simulink library "Microgrid_Library"  (R2020a)
settings: Solver=VariableStepAuto  StartTime=0.0  StopTime=10.0  RelTol=1e-3
libraries: simulink x6944, powerlib x1090, simulink_extras x40, powerlib_meascontrol x20
(part 2 of 77)

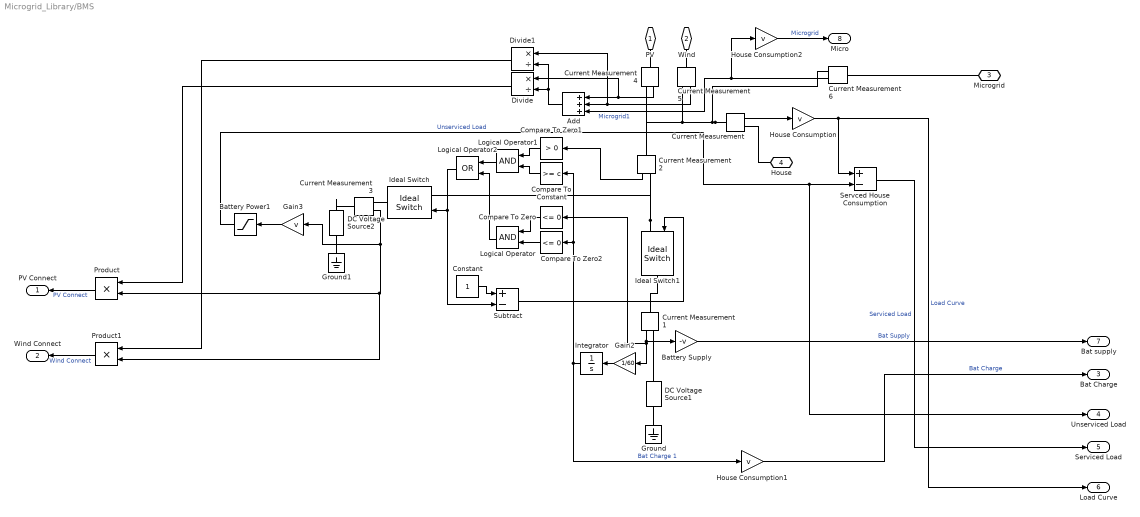
== system Microgrid_Library/BMS ==
  Add : Sum  Inputs=+++
  Battery Power1 : Saturate  UpperLimit=inf  LowerLimit=0
  Battery Supply : Gain  Gain=-v
  Compare To Constant : simulink/Logic and Bit Operations/Compare To Constant  relop=>=  const=c  OutDataTypeStr=boolean
  Compare To Zero : simulink/Logic and Bit Operations/Compare To Zero  relop=<=  OutDataTypeStr=boolean
  Compare To Zero1 : simulink/Logic and Bit Operations/Compare To Zero  relop=>  OutDataTypeStr=boolean
  Compare To Zero2 : simulink/Logic and Bit Operations/Compare To Zero  relop=<=  OutDataTypeStr=boolean
  Constant : Constant
  Current Measurement{,1..6} (7 alike) : powerlib/Measurements/Current Measurement  PhasorSimulation=off  OutputType=Complex  PSBequivalent=0
  DC Voltage Source1 : powerlib/Electrical Sources/DC Voltage Source  Amplitude=v  Measurements=None
  DC Voltage Source2 : powerlib/Electrical Sources/DC Voltage Source  Amplitude=v  Measurements=None
  Divide : Product  Inputs=*/
  Divide1 : Product  Inputs=*/
  Gain2 : Gain  Gain=1/60
  Gain3 : Gain  Gain=v
  Ground : powerlib/Elements/Ground  LConnTagsString=a
  Ground1 : powerlib/Elements/Ground  LConnTagsString=a
  House Consumption : Gain  Gain=v
  House Consumption1 : Gain  Gain=v
  House Consumption2 : Gain  Gain=v
  Ideal Switch : powerlib/Power Electronics/Ideal Switch  Ron=0.001  Lon=0  IC=0  Rs=1e5  Cs=inf  Measurements=off
  Ideal Switch1 : powerlib/Power Electronics/Ideal Switch  Ron=0.001  Lon=0  IC=0  Rs=1e5  Cs=inf  Measurements=off
  Integrator : Integrator  InitialCondition=c  LimitOutput=on  UpperSaturationLimit=c  LowerSaturationLimit=0
  Logical Operator : Logic  AllPortsSameDT=off  OutDataTypeStr=boolean
  Logical Operator1 : Logic  AllPortsSameDT=off  OutDataTypeStr=boolean
  Logical Operator2 : Logic  Operator=OR  AllPortsSameDT=off  OutDataTypeStr=boolean
  Product : Product
  Product1 : Product
  Servced House Consumption : Sum  Inputs=+-
  Subtract : Sum  Inputs=+-
  PV : PMIOPort  Side=Right
  Wind : PMIOPort  Port=2  Side=Right
  Microgrid : PMIOPort  Port=3  Side=Right
  House : PMIOPort  Port=4  Side=Right
  PV Connect : Outport  VectorParamsAs1DForOutWhenUnconnected=off
  Wind Connect : Outport  Port=2  VectorParamsAs1DForOutWhenUnconnected=off
  Bat Charge : Outport  Port=3  VectorParamsAs1DForOutWhenUnconnected=off
  Unserviced Load : Outport  Port=4  VectorParamsAs1DForOutWhenUnconnected=off
  Serviced Load : Outport  Port=5  VectorParamsAs1DForOutWhenUnconnected=off
  Load Curve : Outport  Port=6  VectorParamsAs1DForOutWhenUnconnected=off
  Bat supply : Outport  Port=7  VectorParamsAs1DForOutWhenUnconnected=off
  Micro : Outport  Port=8  VectorParamsAs1DForOutWhenUnconnected=off
  wires:
  House Consumption2/1 -> Micro/1  "Microgrid"
  Current Measurement6/1 -> House Consumption2/1, Add/3  "Microgrid1"
  Divide1/1 -> Product1/1
  Product1/1 -> Wind Connect/1  "Wind Connect"
  Divide/1 -> Product/1
  Product/1 -> PV Connect/1  "PV Connect"
  Add/1 -> Divide1/2, Divide/2
  Current Measurement4/1 -> Add/1, Divide/1
  Current Measurement5/1 -> Divide1/1, Add/2
  House Consumption1/1 -> Bat Charge/1  "Bat Charge"
  Battery Supply/1 -> Bat supply/1  "Bat Supply"
  Servced House Consumption/1 -> Serviced Load/1  "Serviced Load"
  House Consumption/1 -> Servced House Consumption/1, Load Curve/1  "Load Curve"
  Current Measurement/1 -> House Consumption/1
  Battery Power1/1 -> Servced House Consumption/2, Unserviced Load/1  "Unserviced Load"
  Gain3/1 -> Battery Power1/1
  Current Measurement3/1 -> Gain3/1, Product/2, Product1/2
  Integrator/1 -> Compare To Zero2/1, Compare To Constant/1, House Consumption1/1  "Bat Charge 1"
  Gain2/1 -> Integrator/1
  Logical Operator2/1 -> Subtract/2, Ideal Switch/1
  Logical Operator/1 -> Logical Operator2/2
  Logical Operator1/1 -> Logical Operator2/1
  Compare To Constant/1 -> Logical Operator1/2
  Compare To Zero1/1 -> Logical Operator1/1
  Current Measurement2/1 -> Compare To Zero1/1
  Subtract/1 -> Ideal Switch1/1
  Constant/1 -> Subtract/1
  Compare To Zero2/1 -> Logical Operator/2
  Compare To Zero/1 -> Logical Operator/1
  Current Measurement1/1 -> Battery Supply/1, Compare To Zero/1, Gain2/1
  connections:
  Current Measurement6/LConn1 -- Microgrid/RConn1
  House/RConn1 -- Current Measurement/RConn1
  Wind/RConn1 -- Current Measurement5/LConn1
  Current Measurement4/LConn1 -- PV/RConn1
  Current Measurement3/RConn1 -- Ideal Switch/RConn1
  DC Voltage Source2/LConn1 -- Ground1/LConn1
  Current Measurement/LConn1 -- Current Measurement6/RConn1 -- Current Measurement5/RConn1 -- Current Measurement4/RConn1 -- Current Measurement2/LConn1
  Ideal Switch1/LConn1 -- Ideal Switch/LConn1 -- Current Measurement2/RConn1
  Ideal Switch1/RConn1 -- Current Measurement1/LConn1
  DC Voltage Source2/RConn1 -- Current Measurement3/LConn1
  Current Measurement1/RConn1 -- DC Voltage Source1/RConn1
  DC Voltage Source1/LConn1 -- Ground/LConn1

== system Microgrid_Library/Grid ==
  Current Measurement : powerlib/Measurements/Current Measurement  PhasorSimulation=off  OutputType=Complex  PSBequivalent=0
  DC Voltage Source1 : powerlib/Electrical Sources/DC Voltage Source  Amplitude=v  Measurements=None
  Ground : powerlib/Elements/Ground  LConnTagsString=a
  Product : Product
  Voltage Measurement : powerlib/Measurements/Voltage Measurement  PhasorSimulation=off  OutputType=Complex  PSBequivalent=0
  + : PMIOPort  Side=Left
  Voltage : Outport  VectorParamsAs1DForOutWhenUnconnected=off
  Power : Outport  Port=2  VectorParamsAs1DForOutWhenUnconnected=off
  Current : Outport  Port=3  VectorParamsAs1DForOutWhenUnconnected=off
  wires:
  Current Measurement/1 -> Product/1, Current/1
  Voltage Measurement/1 -> Product/2, Voltage/1
  Product/1 -> Power/1
  connections:
  Current Measurement/RConn1 -- +/RConn1
  DC Voltage Source1/RConn1 -- Voltage Measurement/LConn1 -- Current Measurement/LConn1
  DC Voltage Source1/LConn1 -- Voltage Measurement/LConn2 -- Ground/LConn1

== system Microgrid_Library/House 1 S ==
  Clock 1 : Subsystem
      mask Display: image('Clock.jpg');
  Clock 2 : Subsystem
      mask Display: image('Clock.jpg');
  Kettle : Subsystem
      mask Display: image('jug.jpg');
  Lamp 1 : Subsystem
      mask Display: image('Lamp_1.jpg');
  Lamp 2 : Subsystem
      mask Display: image('Lamp_1.jpg');
  Lamp 3 : Subsystem
      mask Display: image('Lamp_1.jpg');
  Laptop_Charger : Subsystem
      mask Display: image('Laptop.jpg');
  Light 1 : Subsystem
      mask Display: image('LightBulb.jpg');
  Light 2 : Subsystem
      mask Display: image('LightBulb.jpg');
  Light 3 : Subsystem
      mask Display: image('LightBulb.jpg');
  Light 4 : Subsystem
      mask Display: image('LightBulb.jpg');
  Light 5 : Subsystem
      mask Display: image('LightBulb.jpg');
  Light 6 : Subsystem
      mask Display: image('LightBulb.jpg');
  Oven : Subsystem
      mask Display: image('oven.jpg');
  Phone_Charger : Subsystem
      mask Display: image('Phone.jpg');
  Refrigerator : Subsystem
      mask Display: image('firdge1.JPG');
  Shower : Subsystem
      mask Display: image('Shower.jpg');
  Stereo : Subsystem
      mask Display: image('Stereo.jpg');
  Subsystem : Subsystem
      mask Display: patch([1 1 0 0],[1 0 0 1], [1, .4, .4])
  Subsystem1 : Subsystem
      mask Display: patch([1 1 0 0],[1 0 0 1], [0.6, .8, 1])
  Subsystem2 : Subsystem
      mask Display: patch([1 1 0 0],[1 0 0 1], [0.4, 1, .4])
  Subsystem3 : Subsystem
      mask Display: patch([1 1 0 0],[1 0 0 1], [0.8, 0.42, 0])
  Subsystem4 : Subsystem
      mask Display: patch([1 1 0 0],[1 0 0 1], [0.75, .75, .75])
  Toaster : Subsystem
      mask Display: image('toaster.jpg');
  Tv : Subsystem
      mask Display: image('Tel.jpg');
  Water tank : Subsystem
      mask Display: image('Hot Water.jpg');
  Connection Port : PMIOPort  Side=Left
  wires:
  Shower/1(Out1) -> Water tank/1(Mass flow rate)
  connections:
  Light 1/LConn1 -- Light 5/LConn1 -- Laptop_Charger/RConn1 -- Laptop_Charger/LConn1 -- Phone_Charger/RConn1 -- Phone_Charger/LConn2 -- Phone_Charger/LConn1 -- Light 6/LConn1 -- Lamp 3/LConn1 -- Stereo/LConn1 -- Tv/LConn1 -- Lamp 2/LConn1 -- Clock 2/LConn1 -- Lamp 1/LConn1 -- Clock 1/LConn1 -- Light 4/LConn1 -- Light 3/LConn1 -- Water tank/LConn1 -- Refrigerator/LConn1 -- Kettle/LConn1 -- Toaster/LConn1 -- Light 2/LConn1 -- Connection Port/RConn1 -- Oven/LConn1

== system Microgrid_Library/House 1 W ==
  Clock 1 : Subsystem
      mask Display: image('Clock.jpg');
  Clock 2 : Subsystem
      mask Display: image('Clock.jpg');
  Heater : Subsystem
      mask Display: image('oil_heater.jpg');
  Kettle : Subsystem
      mask Display: image('jug.jpg');
  Lamp 1 : Subsystem
      mask Display: image('Lamp_1.jpg');
  Lamp 2 : Subsystem
      mask Display: image('Lamp_1.jpg');
  Lamp 3 : Subsystem
      mask Display: image('Lamp_1.jpg');
  Laptop_Charger : Subsystem
      mask Display: image('Laptop.jpg');
  Light 1 : Subsystem
      mask Display: image('LightBulb.jpg');
  Light 2 : Subsystem
      mask Display: image('LightBulb.jpg');
  Light 3 : Subsystem
      mask Display: image('LightBulb.jpg');
  Light 4 : Subsystem
      mask Display: image('LightBulb.jpg');
  Light 5 : Subsystem
      mask Display: image('LightBulb.jpg');
  Light 6 : Subsystem
      mask Display: image('LightBulb.jpg');
  Oven : Subsystem
      mask Display: image('oven.jpg');
  Phone_Charger : Subsystem
      mask Display: image('Phone.jpg');
  Refrigerator : Subsystem
      mask Display: image('firdge1.JPG');
  Shower : Subsystem
      mask Display: image('Shower.jpg');
  Stereo : Subsystem
      mask Display: image('Stereo.jpg');
  Subsystem : Subsystem
      mask Display: patch([1 1 0 0],[1 0 0 1], [1, .4, .4])
  Subsystem1 : Subsystem
      mask Display: patch([1 1 0 0],[1 0 0 1], [0.6, .8, 1])
  Subsystem2 : Subsystem
      mask Display: patch([1 1 0 0],[1 0 0 1], [0.4, 1, .4])
  Subsystem3 : Subsystem
      mask Display: patch([1 1 0 0],[1 0 0 1], [0.8, 0.42, 0])
  Subsystem4 : Subsystem
      mask Display: patch([1 1 0 0],[1 0 0 1], [0.75, .75, .75])
  Toaster : Subsystem
      mask Display: image('toaster.jpg');
  Tv : Subsystem
      mask Display: image('Tel.jpg');
  Water tank : Subsystem
      mask Display: image('Hot Water.jpg');
  Connection Port : PMIOPort  Side=Left
  wires:
  Shower/1(Out1) -> Water tank/1(Mass flow rate)
  connections:
  Light 3/LConn1 -- Light 1/LConn1 -- Connection Port/RConn1 -- Oven/LConn1 -- Light 2/LConn1 -- Toaster/LConn1 -- Kettle/LConn1 -- Refrigerator/LConn1 -- Water tank/LConn1 -- Light 5/LConn1 -- Laptop_Charger/RConn1 -- Laptop_Charger/LConn1 -- Phone_Charger/RConn1 -- Phone_Charger/LConn2 -- Phone_Charger/LConn1 -- Heater/LConn1 -- Stereo/LConn1 -- Tv/LConn1 -- Lamp 3/LConn1 -- Light 6/LConn1 -- Lamp 2/LConn1 -- Clock 2/LConn1 -- Lamp 1/LConn1 -- Clock 1/LConn1 -- Light 4/LConn1

== system Microgrid_Library/House 10 S ==
  Clock 1 : Subsystem
      mask Display: image('Clock.jpg');
  Clock 2 : Subsystem
      mask Display: image('Clock.jpg');
  Kettle : Subsystem
      mask Display: image('jug.jpg');
  Lamp 1 : Subsystem
      mask Display: image('Lamp_1.jpg');
  Lamp 2 : Subsystem
      mask Display: image('Lamp_1.jpg');
  Lamp 3 : Subsystem
      mask Display: image('Lamp_1.jpg');
  Laptop_Charger : Subsystem
      mask Display: image('Laptop.jpg');
  Light 1 : Subsystem
      mask Display: image('LightBulb.jpg');
  Light 2 : Subsystem
      mask Display: image('LightBulb.jpg');
  Light 3 : Subsystem
      mask Display: image('LightBulb.jpg');
  Light 4 : Subsystem
      mask Display: image('LightBulb.jpg');
  Light 5 : Subsystem
      mask Display: image('LightBulb.jpg');
  Light 6 : Subsystem
      mask Display: image('LightBulb.jpg');
  Oven : Subsystem
      mask Display: image('oven.jpg');
  Phone_Charger : Subsystem
      mask Display: image('Phone.jpg');
  Refrigerator : Subsystem
      mask Display: image('firdge1.JPG');
  Shower : Subsystem
      mask Display: image('Shower.jpg');
  Stereo : Subsystem
      mask Display: image('Stereo.jpg');
  Subsystem : Subsystem
      mask Display: patch([1 1 0 0],[1 0 0 1], [1, .4, .4])
  Subsystem1 : Subsystem
      mask Display: patch([1 1 0 0],[1 0 0 1], [0.6, .8, 1])
  Subsystem2 : Subsystem
      mask Display: patch([1 1 0 0],[1 0 0 1], [0.4, 1, .4])
  Subsystem3 : Subsystem
      mask Display: patch([1 1 0 0],[1 0 0 1], [0.8, 0.42, 0])
  Subsystem4 : Subsystem
      mask Display: patch([1 1 0 0],[1 0 0 1], [0.75, .75, .75])
  Toaster : Subsystem
      mask Display: image('toaster.jpg');
  Tv : Subsystem
      mask Display: image('Tel.jpg');
  Water tank : Subsystem
      mask Display: image('Hot Water.jpg');
  Connection Port : PMIOPort  Side=Left
  wires:
  Shower/1(Out1) -> Water tank/1(Mass flow rate)
  connections:
  Laptop_Charger/LConn1 -- Laptop_Charger/RConn1 -- Lamp 2/LConn1 -- Light 5/LConn1 -- Clock 2/LConn1 -- Lamp 1/LConn1 -- Clock 1/LConn1 -- Light 4/LConn1 -- Light 3/LConn1 -- Water tank/LConn1 -- Refrigerator/LConn1 -- Kettle/LConn1 -- Toaster/LConn1 -- Light 2/LConn1 -- Oven/LConn1 -- Connection Port/RConn1 -- Light 1/LConn1 -- Phone_Charger/RConn1 -- Phone_Charger/LConn2 -- Phone_Charger/LConn1 -- Light 6/LConn1 -- Lamp 3/LConn1 -- Stereo/LConn1 -- Tv/LConn1

== system Microgrid_Library/House 10 W ==
  Clock 1 : Subsystem
      mask Display: image('Clock.jpg');
  Clock 2 : Subsystem
      mask Display: image('Clock.jpg');
  Heater : Subsystem
      mask Display: image('oil_heater.jpg');
  Kettle : Subsystem
      mask Display: image('jug.jpg');
  Lamp 1 : Subsystem
      mask Display: image('Lamp_1.jpg');
  Lamp 2 : Subsystem
      mask Display: image('Lamp_1.jpg');
  Lamp 3 : Subsystem
      mask Display: image('Lamp_1.jpg');
  Laptop_Charger : Subsystem
      mask Display: image('Laptop.jpg');
  Light 1 : Subsystem
      mask Display: image('LightBulb.jpg');
  Light 2 : Subsystem
      mask Display: image('LightBulb.jpg');
  Light 3 : Subsystem
      mask Display: image('LightBulb.jpg');
  Light 4 : Subsystem
      mask Display: image('LightBulb.jpg');
  Light 5 : Subsystem
      mask Display: image('LightBulb.jpg');
  Light 6 : Subsystem
      mask Display: image('LightBulb.jpg');
  Oven : Subsystem
      mask Display: image('oven.jpg');
  Phone_Charger : Subsystem
      mask Display: image('Phone.jpg');
  Refrigerator : Subsystem
      mask Display: image('firdge1.JPG');
  Shower : Subsystem
      mask Display: image('Shower.jpg');
  Stereo : Subsystem
      mask Display: image('Stereo.jpg');
  Subsystem : Subsystem
      mask Display: patch([1 1 0 0],[1 0 0 1], [1, .4, .4])
  Subsystem1 : Subsystem
      mask Display: patch([1 1 0 0],[1 0 0 1], [0.6, .8, 1])
  Subsystem2 : Subsystem
      mask Display: patch([1 1 0 0],[1 0 0 1], [0.4, 1, .4])
  Subsystem3 : Subsystem
      mask Display: patch([1 1 0 0],[1 0 0 1], [0.8, 0.42, 0])
  Subsystem4 : Subsystem
      mask Display: patch([1 1 0 0],[1 0 0 1], [0.75, .75, .75])
  Toaster : Subsystem
      mask Display: image('toaster.jpg');
  Tv : Subsystem
      mask Display: image('Tel.jpg');
  Water tank : Subsystem
      mask Display: image('Hot Water.jpg');
  Connection Port : PMIOPort  Side=Left
  wires:
  Shower/1(Out1) -> Water tank/1(Mass flow rate)
  connections:
  Tv/LConn1 -- Stereo/LConn1 -- Heater/LConn1 -- Lamp 3/LConn1 -- Light 6/LConn1 -- Phone_Charger/LConn1 -- Phone_Charger/LConn2 -- Phone_Charger/RConn1 -- Laptop_Charger/LConn1 -- Laptop_Charger/RConn1 -- Lamp 2/LConn1 -- Light 5/LConn1 -- Clock 2/LConn1 -- Lamp 1/LConn1 -- Clock 1/LConn1 -- Light 4/LConn1 -- Light 1/LConn1 -- Connection Port/RConn1 -- Oven/LConn1 -- Light 2/LConn1 -- Toaster/LConn1 -- Kettle/LConn1 -- Refrigerator/LConn1 -- Water tank/LConn1 -- Light 3/LConn1

== system Microgrid_Library/House 2 S ==
  Clock 1 : Subsystem
      mask Display: image('Clock.jpg');
  Clock 2 : Subsystem
      mask Display: image('Clock.jpg');
  Kettle : Subsystem
      mask Display: image('jug.jpg');
  Lamp 1 : Subsystem
      mask Display: image('Lamp_1.jpg');
  Lamp 2 : Subsystem
      mask Display: image('Lamp_1.jpg');
  Lamp 3 : Subsystem
      mask Display: image('Lamp_1.jpg');
  Laptop_Charger : Subsystem
      mask Display: image('Laptop.jpg');
  Light 1 : Subsystem
      mask Display: image('LightBulb.jpg');
  Light 2 : Subsystem
      mask Display: image('Dishwasher.jpg');
  Light 4 : Subsystem
      mask Display: image('LightBulb.jpg');
  Light 5 : Subsystem
      mask Display: image('LightBulb.jpg');
  Light 6 : Subsystem
      mask Display: image('LightBulb.jpg');
  Light 7 : Subsystem
      mask Display: image('LightBulb.jpg');
  Oven : Subsystem
      mask Display: image('oven.jpg');
  Phone_Charger : Subsystem
      mask Display: image('Phone.jpg');
  Refrigerator : Subsystem
      mask Display: image('firdge1.JPG');
  Shower : Subsystem
      mask Display: image('Shower.jpg');
  Stereo : Subsystem
      mask Display: image('Stereo.jpg');
  Subsystem : Subsystem
      mask Display: patch([1 1 0 0],[1 0 0 1], [1, .4, .4])
  Subsystem1 : Subsystem
      mask Display: patch([1 1 0 0],[1 0 0 1], [0.6, .8, 1])
  Subsystem2 : Subsystem
      mask Display: patch([1 1 0 0],[1 0 0 1], [0.4, 1, .4])
  Subsystem3 : Subsystem
      mask Display: patch([1 1 0 0],[1 0 0 1], [0.8, 0.42, 0])
  Subsystem4 : Subsystem
      mask Display: patch([1 1 0 0],[1 0 0 1], [0.75, .75, .75])
  Toaster : Subsystem
      mask Display: image('toaster.jpg');
  Tv : Subsystem
      mask Display: image('Tel.jpg');
  Water tank : Subsystem
      mask Display: image('Hot Water.jpg');
  Connection Port : PMIOPort  Side=Left
  wires:
  Shower/1(Out1) -> Water tank/1(Mass flow rate)
  connections:
  Tv/LConn1 -- Stereo/LConn1 -- Lamp 3/LConn1 -- Light 7/LConn1 -- Phone_Charger/LConn1 -- Phone_Charger/LConn2 -- Phone_Charger/RConn1 -- Kettle/LConn1 -- Toaster/LConn1 -- Light 1/LConn1 -- Oven/LConn1 -- Connection Port/RConn1 -- Light 2/LConn1 -- Refrigerator/LConn1 -- Water tank/LConn1 -- Light 4/LConn1 -- Light 5/LConn1 -- Clock 1/LConn1 -- Lamp 1/LConn1 -- Clock 2/LConn1 -- Light 6/LConn1 -- Lamp 2/LConn1 -- Laptop_Charger/RConn1 -- Laptop_Charger/LConn1

== system Microgrid_Library/House 2 W ==
  Clock 1 : Subsystem
      mask Display: image('Clock.jpg');
  Clock 2 : Subsystem
      mask Display: image('Clock.jpg');
  Heater : Subsystem
      mask Display: image('oil_heater.jpg');
  Kettle : Subsystem
      mask Display: image('jug.jpg');
  Lamp 1 : Subsystem
      mask Display: image('Lamp_1.jpg');
  Lamp 2 : Subsystem
      mask Display: image('Lamp_1.jpg');
  Lamp 3 : Subsystem
      mask Display: image('Lamp_1.jpg');
  Laptop_Charger : Subsystem
      mask Display: image('Laptop.jpg');
  Light 1 : Subsystem
      mask Display: image('LightBulb.jpg');
  Light 2 : Subsystem
      mask Display: image('Dishwasher.jpg');
  Light 4 : Subsystem
      mask Display: image('LightBulb.jpg');
  Light 5 : Subsystem
      mask Display: image('LightBulb.jpg');
  Light 6 : Subsystem
      mask Display: image('LightBulb.jpg');
  Light 7 : Subsystem
      mask Display: image('LightBulb.jpg');
  Oven : Subsystem
      mask Display: image('oven.jpg');
  Phone_Charger : Subsystem
      mask Display: image('Phone.jpg');
  Refrigerator : Subsystem
      mask Display: image('firdge1.JPG');
  Shower : Subsystem
      mask Display: image('Shower.jpg');
  Stereo : Subsystem
      mask Display: image('Stereo.jpg');
  Subsystem : Subsystem
      mask Display: patch([1 1 0 0],[1 0 0 1], [1, .4, .4])
  Subsystem1 : Subsystem
      mask Display: patch([1 1 0 0],[1 0 0 1], [0.6, .8, 1])
  Subsystem2 : Subsystem
      mask Display: patch([1 1 0 0],[1 0 0 1], [0.4, 1, .4])
  Subsystem3 : Subsystem
      mask Display: patch([1 1 0 0],[1 0 0 1], [0.8, 0.42, 0])
  Subsystem4 : Subsystem
      mask Display: patch([1 1 0 0],[1 0 0 1], [0.75, .75, .75])
  Toaster : Subsystem
      mask Display: image('toaster.jpg');
  Tv : Subsystem
      mask Display: image('Tel.jpg');
  Water tank : Subsystem
      mask Display: image('Hot Water.jpg');
  Connection Port : PMIOPort  Side=Left
  wires:
  Shower/1(Out1) -> Water tank/1(Mass flow rate)
  connections:
  Connection Port/RConn1 -- Oven/LConn1 -- Light 1/LConn1 -- Heater/LConn1 -- Tv/LConn1 -- Stereo/LConn1 -- Lamp 3/LConn1 -- Light 7/LConn1 -- Phone_Charger/LConn1 -- Phone_Charger/LConn2 -- Phone_Charger/RConn1 -- Laptop_Charger/LConn1 -- Laptop_Charger/RConn1 -- Lamp 2/LConn1 -- Light 6/LConn1 -- Clock 2/LConn1 -- Lamp 1/LConn1 -- Clock 1/LConn1 -- Light 5/LConn1 -- Light 4/LConn1 -- Water tank/LConn1 -- Refrigerator/LConn1 -- Kettle/LConn1 -- Light 2/LConn1 -- Toaster/LConn1

== system Microgrid_Library/House 3 S ==
  Clock 1 : Subsystem
      mask Display: image('Clock.jpg');
  Clock 2 : Subsystem
      mask Display: image('Clock.jpg');
  Kettle : Subsystem
      mask Display: image('jug.jpg');
  Lamp 1 : Subsystem
      mask Display: image('Lamp_1.jpg');
  Lamp 2 : Subsystem
      mask Display: image('Lamp_1.jpg');
  Lamp 3 : Subsystem
      mask Display: image('Lamp_1.jpg');
  Laptop_Charger : Subsystem
      mask Display: image('Laptop.jpg');
  Light 1 : Subsystem
      mask Display: image('LightBulb.jpg');
  Light 2 : Subsystem
      mask Display: image('LightBulb.jpg');
  Light 3 : Subsystem
      mask Display: image('LightBulb.jpg');
  Light 4 : Subsystem
      mask Display: image('LightBulb.jpg');
  Light 5 : Subsystem
      mask Display: image('LightBulb.jpg');
  Light 6 : Subsystem
      mask Display: image('LightBulb.jpg');
  Oven : Subsystem
      mask Display: image('oven.jpg');
  Phone_Charger : Subsystem
      mask Display: image('Phone.jpg');
  Refrigerator : Subsystem
      mask Display: image('firdge1.JPG');
  Shower : Subsystem
      mask Display: image('Shower.jpg');
  Stereo : Subsystem
      mask Display: image('Stereo.jpg');
  Subsystem : Subsystem
      mask Display: patch([1 1 0 0],[1 0 0 1], [1, .4, .4])
  Subsystem1 : Subsystem
      mask Display: patch([1 1 0 0],[1 0 0 1], [0.6, .8, 1])
  Subsystem2 : Subsystem
      mask Display: patch([1 1 0 0],[1 0 0 1], [0.4, 1, .4])
  Subsystem3 : Subsystem
      mask Display: patch([1 1 0 0],[1 0 0 1], [0.8, 0.42, 0])
  Subsystem4 : Subsystem
      mask Display: patch([1 1 0 0],[1 0 0 1], [0.75, .75, .75])
  Toaster : Subsystem
      mask Display: image('toaster.jpg');
  Tv : Subsystem
      mask Display: image('Tel.jpg');
  Water tank : Subsystem
      mask Display: image('Hot Water.jpg');
  Connection Port : PMIOPort  Side=Left
  wires:
  Shower/1(Out1) -> Water tank/1(Mass flow rate)
  connections:
  Laptop_Charger/LConn1 -- Laptop_Charger/RConn1 -- Lamp 2/LConn1 -- Light 5/LConn1 -- Clock 2/LConn1 -- Lamp 1/LConn1 -- Clock 1/LConn1 -- Light 4/LConn1 -- Light 3/LConn1 -- Water tank/LConn1 -- Refrigerator/LConn1 -- Kettle/LConn1 -- Toaster/LConn1 -- Light 2/LConn1 -- Oven/LConn1 -- Connection Port/RConn1 -- Light 1/LConn1 -- Phone_Charger/RConn1 -- Phone_Charger/LConn2 -- Phone_Charger/LConn1 -- Light 6/LConn1 -- Lamp 3/LConn1 -- Stereo/LConn1 -- Tv/LConn1

== system Microgrid_Library/House 3 W ==
  Clock 1 : Subsystem
      mask Display: image('Clock.jpg');
  Clock 2 : Subsystem
      mask Display: image('Clock.jpg');
  Heater : Subsystem
      mask Display: image('oil_heater.jpg');
  Kettle : Subsystem
      mask Display: image('jug.jpg');
  Lamp 1 : Subsystem
      mask Display: image('Lamp_1.jpg');
  Lamp 2 : Subsystem
      mask Display: image('Lamp_1.jpg');
  Lamp 3 : Subsystem
      mask Display: image('Lamp_1.jpg');
  Laptop_Charger : Subsystem
      mask Display: image('Laptop.jpg');
  Light 1 : Subsystem
      mask Display: image('LightBulb.jpg');
  Light 2 : Subsystem
      mask Display: image('LightBulb.jpg');
  Light 3 : Subsystem
      mask Display: image('LightBulb.jpg');
  Light 4 : Subsystem
      mask Display: image('LightBulb.jpg');
  Light 5 : Subsystem
      mask Display: image('LightBulb.jpg');
  Light 6 : Subsystem
      mask Display: image('LightBulb.jpg');
  Oven : Subsystem
      mask Display: image('oven.jpg');
  Phone_Charger : Subsystem
      mask Display: image('Phone.jpg');
  Refrigerator : Subsystem
      mask Display: image('firdge1.JPG');
  Shower : Subsystem
      mask Display: image('Shower.jpg');
  Stereo : Subsystem
      mask Display: image('Stereo.jpg');
  Subsystem : Subsystem
      mask Display: patch([1 1 0 0],[1 0 0 1], [1, .4, .4])
  Subsystem1 : Subsystem
      mask Display: patch([1 1 0 0],[1 0 0 1], [0.6, .8, 1])
  Subsystem2 : Subsystem
      mask Display: patch([1 1 0 0],[1 0 0 1], [0.4, 1, .4])
  Subsystem3 : Subsystem
      mask Display: patch([1 1 0 0],[1 0 0 1], [0.8, 0.42, 0])
  Subsystem4 : Subsystem
      mask Display: patch([1 1 0 0],[1 0 0 1], [0.75, .75, .75])
  Toaster : Subsystem
      mask Display: image('toaster.jpg');
  Tv : Subsystem
      mask Display: image('Tel.jpg');
  Water tank : Subsystem
      mask Display: image('Hot Water.jpg');
  Connection Port : PMIOPort  Side=Left
  wires:
  Shower/1(Out1) -> Water tank/1(Mass flow rate)
  connections:
  Tv/LConn1 -- Stereo/LConn1 -- Heater/LConn1 -- Lamp 3/LConn1 -- Light 6/LConn1 -- Phone_Charger/LConn1 -- Phone_Charger/LConn2 -- Phone_Charger/RConn1 -- Laptop_Charger/LConn1 -- Laptop_Charger/RConn1 -- Lamp 2/LConn1 -- Light 5/LConn1 -- Clock 2/LConn1 -- Lamp 1/LConn1 -- Clock 1/LConn1 -- Light 4/LConn1 -- Light 1/LConn1 -- Connection Port/RConn1 -- Oven/LConn1 -- Light 2/LConn1 -- Toaster/LConn1 -- Kettle/LConn1 -- Refrigerator/LConn1 -- Water tank/LConn1 -- Light 3/LConn1

== system Microgrid_Library/House 4 S ==
  Clock 1 : Subsystem
      mask Display: image('Clock.jpg');
  Clock 2 : Subsystem
      mask Display: image('Clock.jpg');
  Kettle : Subsystem
      mask Display: image('jug.jpg');
  Lamp 1 : Subsystem
      mask Display: image('Lamp_1.jpg');
  Lamp 2 : Subsystem
      mask Display: image('Lamp_1.jpg');
  Lamp 3 : Subsystem
      mask Display: image('Lamp_1.jpg');
  Laptop_Charger : Subsystem
      mask Display: image('Laptop.jpg');
  Light 1 : Subsystem
      mask Display: image('LightBulb.jpg');
  Light 2 : Subsystem
      mask Display: image('LightBulb.jpg');
  Light 3 : Subsystem
      mask Display: image('LightBulb.jpg');
  Light 4 : Subsystem
      mask Display: image('LightBulb.jpg');
  Light 5 : Subsystem
      mask Display: image('LightBulb.jpg');
  Light 6 : Subsystem
      mask Display: image('LightBulb.jpg');
  Oven : Subsystem
      mask Display: image('oven.jpg');
  Phone_Charger : Subsystem
      mask Display: image('Phone.jpg');
  Refrigerator : Subsystem
      mask Display: image('firdge1.JPG');
  Shower : Subsystem
      mask Display: image('Shower.jpg');
  Stereo : Subsystem
      mask Display: image('Stereo.jpg');
  Subsystem : Subsystem
      mask Display: patch([1 1 0 0],[1 0 0 1], [1, .4, .4])
  Subsystem1 : Subsystem
      mask Display: patch([1 1 0 0],[1 0 0 1], [0.6, .8, 1])
  Subsystem2 : Subsystem
      mask Display: patch([1 1 0 0],[1 0 0 1], [0.4, 1, .4])
  Subsystem3 : Subsystem
      mask Display: patch([1 1 0 0],[1 0 0 1], [0.8, 0.42, 0])
  Subsystem4 : Subsystem
      mask Display: patch([1 1 0 0],[1 0 0 1], [0.75, .75, .75])
  Toaster : Subsystem
      mask Display: image('toaster.jpg');
  Tv : Subsystem
      mask Display: image('Tel.jpg');
  Water tank : Subsystem
      mask Display: image('Hot Water.jpg');
  Connection Port : PMIOPort  Side=Left
  wires:
  Shower/1(Out1) -> Water tank/1(Mass flow rate)
  connections:
  Tv/LConn1 -- Stereo/LConn1 -- Lamp 3/LConn1 -- Light 6/LConn1 -- Phone_Charger/LConn1 -- Phone_Charger/LConn2 -- Phone_Charger/RConn1 -- Water tank/LConn1 -- Light 1/LConn1 -- Connection Port/RConn1 -- Oven/LConn1 -- Light 2/LConn1 -- Toaster/LConn1 -- Kettle/LConn1 -- Refrigerator/LConn1 -- Light 3/LConn1 -- Light 4/LConn1 -- Clock 1/LConn1 -- Lamp 1/LConn1 -- Clock 2/LConn1 -- Light 5/LConn1 -- Lamp 2/LConn1 -- Laptop_Charger/RConn1 -- Laptop_Charger/LConn1

== system Microgrid_Library/House 4 W ==
  Clock 1 : Subsystem
      mask Display: image('Clock.jpg');
  Clock 2 : Subsystem
      mask Display: image('Clock.jpg');
  Heater : Subsystem
      mask Display: image('oil_heater.jpg');
  Kettle : Subsystem
      mask Display: image('jug.jpg');
  Lamp 1 : Subsystem
      mask Display: image('Lamp_1.jpg');
  Lamp 2 : Subsystem
      mask Display: image('Lamp_1.jpg');
  Lamp 3 : Subsystem
      mask Display: image('Lamp_1.jpg');
  Laptop_Charger : Subsystem
      mask Display: image('Laptop.jpg');
  Light 1 : Subsystem
      mask Display: image('LightBulb.jpg');
  Light 2 : Subsystem
      mask Display: image('LightBulb.jpg');
  Light 3 : Subsystem
      mask Display: image('LightBulb.jpg');
  Light 4 : Subsystem
      mask Display: image('LightBulb.jpg');
  Light 5 : Subsystem
      mask Display: image('LightBulb.jpg');
  Light 6 : Subsystem
      mask Display: image('LightBulb.jpg');
  Oven : Subsystem
      mask Display: image('oven.jpg');
  Phone_Charger : Subsystem
      mask Display: image('Phone.jpg');
  Refrigerator : Subsystem
      mask Display: image('firdge1.JPG');
  Shower : Subsystem
      mask Display: image('Shower.jpg');
  Stereo : Subsystem
      mask Display: image('Stereo.jpg');
  Subsystem : Subsystem
      mask Display: patch([1 1 0 0],[1 0 0 1], [1, .4, .4])
  Subsystem1 : Subsystem
      mask Display: patch([1 1 0 0],[1 0 0 1], [0.6, .8, 1])
  Subsystem2 : Subsystem
      mask Display: patch([1 1 0 0],[1 0 0 1], [0.4, 1, .4])
  Subsystem3 : Subsystem
      mask Display: patch([1 1 0 0],[1 0 0 1], [0.8, 0.42, 0])
  Subsystem4 : Subsystem
      mask Display: patch([1 1 0 0],[1 0 0 1], [0.75, .75, .75])
  Toaster : Subsystem
      mask Display: image('toaster.jpg');
  Tv : Subsystem
      mask Display: image('Tel.jpg');
  Water tank : Subsystem
      mask Display: image('Hot Water.jpg');
  Connection Port : PMIOPort  Side=Left
  wires:
  Shower/1(Out1) -> Water tank/1(Mass flow rate)
  connections:
  Light 3/LConn1 -- Water tank/LConn1 -- Refrigerator/LConn1 -- Kettle/LConn1 -- Toaster/LConn1 -- Light 2/LConn1 -- Oven/LConn1 -- Connection Port/RConn1 -- Light 1/LConn1 -- Light 4/LConn1 -- Clock 1/LConn1 -- Lamp 1/LConn1 -- Clock 2/LConn1 -- Light 5/LConn1 -- Lamp 2/LConn1 -- Laptop_Charger/RConn1 -- Laptop_Charger/LConn1 -- Phone_Charger/RConn1 -- Phone_Charger/LConn2 -- Phone_Charger/LConn1 -- Light 6/LConn1 -- Lamp 3/LConn1 -- Heater/LConn1 -- Stereo/LConn1 -- Tv/LConn1

== system Microgrid_Library/House 5 S   ==
  Clock 1 : Subsystem
      mask Display: image('Clock.jpg');
  Clock 2 : Subsystem
      mask Display: image('Clock.jpg');
  Kettle : Subsystem
      mask Display: image('jug.jpg');
  Lamp 1 : Subsystem
      mask Display: image('Lamp_1.jpg');
  Lamp 2 : Subsystem
      mask Display: image('Lamp_1.jpg');
  Lamp 3 : Subsystem
      mask Display: image('Lamp_1.jpg');
  Laptop_Charger : Subsystem
      mask Display: image('Laptop.jpg');
  Light 1 : Subsystem
      mask Display: image('LightBulb.jpg');
  Light 2 : Subsystem
      mask Display: image('Dishwasher.jpg');
  Light 4 : Subsystem
      mask Display: image('LightBulb.jpg');
  Light 5 : Subsystem
      mask Display: image('LightBulb.jpg');
  Light 6 : Subsystem
      mask Display: image('LightBulb.jpg');
  Light 7 : Subsystem
      mask Display: image('LightBulb.jpg');
  Oven : Subsystem
      mask Display: image('oven.jpg');
  Phone_Charger : Subsystem
      mask Display: image('Phone.jpg');
  Refrigerator : Subsystem
      mask Display: image('firdge1.JPG');
  Shower : Subsystem
      mask Display: image('Shower.jpg');
  Stereo : Subsystem
      mask Display: image('Stereo.jpg');
  Subsystem : Subsystem
      mask Display: patch([1 1 0 0],[1 0 0 1], [1, .4, .4])
  Subsystem1 : Subsystem
      mask Display: patch([1 1 0 0],[1 0 0 1], [0.6, .8, 1])
  Subsystem2 : Subsystem
      mask Display: patch([1 1 0 0],[1 0 0 1], [0.4, 1, .4])
  Subsystem3 : Subsystem
      mask Display: patch([1 1 0 0],[1 0 0 1], [0.8, 0.42, 0])
  Subsystem4 : Subsystem
      mask Display: patch([1 1 0 0],[1 0 0 1], [0.75, .75, .75])
  Toaster : Subsystem
      mask Display: image('toaster.jpg');
  Tv : Subsystem
      mask Display: image('Tel.jpg');
  Water tank : Subsystem
      mask Display: image('Hot Water.jpg');
  Connection Port : PMIOPort  Side=Left
  wires:
  Shower/1(Out1) -> Water tank/1(Mass flow rate)
  connections:
  Lamp 2/LConn1 -- Light 6/LConn1 -- Clock 2/LConn1 -- Lamp 1/LConn1 -- Clock 1/LConn1 -- Light 5/LConn1 -- Light 4/LConn1 -- Water tank/LConn1 -- Refrigerator/LConn1 -- Light 2/LConn1 -- Connection Port/RConn1 -- Oven/LConn1 -- Light 1/LConn1 -- Toaster/LConn1 -- Kettle/LConn1 -- Laptop_Charger/RConn1 -- Laptop_Charger/LConn1 -- Phone_Charger/RConn1 -- Phone_Charger/LConn2 -- Phone_Charger/LConn1 -- Light 7/LConn1 -- Lamp 3/LConn1 -- Stereo/LConn1 -- Tv/LConn1

== system Microgrid_Library/House 5 W ==
  Clock 1 : Subsystem
      mask Display: image('Clock.jpg');
  Clock 2 : Subsystem
      mask Display: image('Clock.jpg');
  Heater : Subsystem
      mask Display: image('oil_heater.jpg');
  Kettle : Subsystem
      mask Display: image('jug.jpg');
  Lamp 1 : Subsystem
      mask Display: image('Lamp_1.jpg');
  Lamp 2 : Subsystem
      mask Display: image('Lamp_1.jpg');
  Lamp 3 : Subsystem
      mask Display: image('Lamp_1.jpg');
  Laptop_Charger : Subsystem
      mask Display: image('Laptop.jpg');
  Light 1 : Subsystem
      mask Display: image('LightBulb.jpg');
  Light 2 : Subsystem
      mask Display: image('Dishwasher.jpg');
  Light 4 : Subsystem
      mask Display: image('LightBulb.jpg');
  Light 5 : Subsystem
      mask Display: image('LightBulb.jpg');
  Light 6 : Subsystem
      mask Display: image('LightBulb.jpg');
  Light 7 : Subsystem
      mask Display: image('LightBulb.jpg');
  Oven : Subsystem
      mask Display: image('oven.jpg');
  Phone_Charger : Subsystem
      mask Display: image('Phone.jpg');
  Refrigerator : Subsystem
      mask Display: image('firdge1.JPG');
  Shower : Subsystem
      mask Display: image('Shower.jpg');
  Stereo : Subsystem
      mask Display: image('Stereo.jpg');
  Subsystem : Subsystem
      mask Display: patch([1 1 0 0],[1 0 0 1], [1, .4, .4])
  Subsystem1 : Subsystem
      mask Display: patch([1 1 0 0],[1 0 0 1], [0.6, .8, 1])
  Subsystem2 : Subsystem
      mask Display: patch([1 1 0 0],[1 0 0 1], [0.4, 1, .4])
  Subsystem3 : Subsystem
      mask Display: patch([1 1 0 0],[1 0 0 1], [0.8, 0.42, 0])
  Subsystem4 : Subsystem
      mask Display: patch([1 1 0 0],[1 0 0 1], [0.75, .75, .75])
  Toaster : Subsystem
      mask Display: image('toaster.jpg');
  Tv : Subsystem
      mask Display: image('Tel.jpg');
  Water tank : Subsystem
      mask Display: image('Hot Water.jpg');
  Connection Port : PMIOPort  Side=Left
  wires:
  Shower/1(Out1) -> Water tank/1(Mass flow rate)
  connections:
  Connection Port/RConn1 -- Oven/LConn1 -- Light 1/LConn1 -- Toaster/LConn1 -- Light 2/LConn1 -- Kettle/LConn1 -- Heater/LConn1 -- Tv/LConn1 -- Stereo/LConn1 -- Lamp 3/LConn1 -- Light 7/LConn1 -- Phone_Charger/LConn1 -- Phone_Charger/LConn2 -- Phone_Charger/RConn1 -- Laptop_Charger/LConn1 -- Laptop_Charger/RConn1 -- Lamp 2/LConn1 -- Light 6/LConn1 -- Clock 2/LConn1 -- Lamp 1/LConn1 -- Clock 1/LConn1 -- Light 5/LConn1 -- Light 4/LConn1 -- Water tank/LConn1 -- Refrigerator/LConn1

== system Microgrid_Library/House 6 S ==
  Clock 1 : Subsystem
      mask Display: image('Clock.jpg');
  Clock 2 : Subsystem
      mask Display: image('Clock.jpg');
  Kettle : Subsystem
      mask Display: image('jug.jpg');
  Lamp 1 : Subsystem
      mask Display: image('Lamp_1.jpg');
  Lamp 2 : Subsystem
      mask Display: image('Lamp_1.jpg');
  Lamp 3 : Subsystem
      mask Display: image('Lamp_1.jpg');
  Laptop_Charger : Subsystem
      mask Display: image('Laptop.jpg');
  Light 1 : Subsystem
      mask Display: image('LightBulb.jpg');
  Light 2 : Subsystem
      mask Display: image('Dishwasher.jpg');
  Light 4 : Subsystem
      mask Display: image('LightBulb.jpg');
  Light 5 : Subsystem
      mask Display: image('LightBulb.jpg');
  Light 6 : Subsystem
      mask Display: image('LightBulb.jpg');
  Light 7 : Subsystem
      mask Display: image('LightBulb.jpg');
  Oven : Subsystem
      mask Display: image('oven.jpg');
  Phone_Charger : Subsystem
      mask Display: image('Phone.jpg');
  Refrigerator : Subsystem
      mask Display: image('firdge1.JPG');
  Shower : Subsystem
      mask Display: image('Shower.jpg');
  Stereo : Subsystem
      mask Display: image('Stereo.jpg');
  Subsystem : Subsystem
      mask Display: patch([1 1 0 0],[1 0 0 1], [1, .4, .4])
  Subsystem1 : Subsystem
      mask Display: patch([1 1 0 0],[1 0 0 1], [0.6, .8, 1])
  Subsystem2 : Subsystem
      mask Display: patch([1 1 0 0],[1 0 0 1], [0.4, 1, .4])
  Subsystem3 : Subsystem
      mask Display: patch([1 1 0 0],[1 0 0 1], [0.8, 0.42, 0])
  Subsystem4 : Subsystem
      mask Display: patch([1 1 0 0],[1 0 0 1], [0.75, .75, .75])
  Toaster : Subsystem
      mask Display: image('toaster.jpg');
  Tv : Subsystem
      mask Display: image('Tel.jpg');
  Water tank : Subsystem
      mask Display: image('Hot Water.jpg');
  Connection Port : PMIOPort  Side=Left
  wires:
  Shower/1(Out1) -> Water tank/1(Mass flow rate)
  connections:
  Lamp 2/LConn1 -- Light 6/LConn1 -- Clock 2/LConn1 -- Lamp 1/LConn1 -- Clock 1/LConn1 -- Light 5/LConn1 -- Light 4/LConn1 -- Water tank/LConn1 -- Refrigerator/LConn1 -- Light 2/LConn1 -- Connection Port/RConn1 -- Oven/LConn1 -- Light 1/LConn1 -- Toaster/LConn1 -- Kettle/LConn1 -- Laptop_Charger/RConn1 -- Laptop_Charger/LConn1 -- Phone_Charger/RConn1 -- Phone_Charger/LConn2 -- Phone_Charger/LConn1 -- Light 7/LConn1 -- Lamp 3/LConn1 -- Stereo/LConn1 -- Tv/LConn1

== system Microgrid_Library/House 6 W ==
  Clock 1 : Subsystem
      mask Display: image('Clock.jpg');
  Clock 2 : Subsystem
      mask Display: image('Clock.jpg');
  Heater : Subsystem
      mask Display: image('oil_heater.jpg');
  Kettle : Subsystem
      mask Display: image('jug.jpg');
  Lamp 1 : Subsystem
      mask Display: image('Lamp_1.jpg');
  Lamp 2 : Subsystem
      mask Display: image('Lamp_1.jpg');
  Lamp 3 : Subsystem
      mask Display: image('Lamp_1.jpg');
  Laptop_Charger : Subsystem
      mask Display: image('Laptop.jpg');
  Light 1 : Subsystem
      mask Display: image('LightBulb.jpg');
  Light 2 : Subsystem
      mask Display: image('Dishwasher.jpg');
  Light 4 : Subsystem
      mask Display: image('LightBulb.jpg');
  Light 5 : Subsystem
      mask Display: image('LightBulb.jpg');
  Light 6 : Subsystem
      mask Display: image('LightBulb.jpg');
  Light 7 : Subsystem
      mask Display: image('LightBulb.jpg');
  Oven : Subsystem
      mask Display: image('oven.jpg');
  Phone_Charger : Subsystem
      mask Display: image('Phone.jpg');
  Refrigerator : Subsystem
      mask Display: image('firdge1.JPG');
  Shower : Subsystem
      mask Display: image('Shower.jpg');
  Stereo : Subsystem
      mask Display: image('Stereo.jpg');
  Subsystem : Subsystem
      mask Display: patch([1 1 0 0],[1 0 0 1], [1, .4, .4])
  Subsystem1 : Subsystem
      mask Display: patch([1 1 0 0],[1 0 0 1], [0.6, .8, 1])
  Subsystem2 : Subsystem
      mask Display: patch([1 1 0 0],[1 0 0 1], [0.4, 1, .4])
  Subsystem3 : Subsystem
      mask Display: patch([1 1 0 0],[1 0 0 1], [0.8, 0.42, 0])
  Subsystem4 : Subsystem
      mask Display: patch([1 1 0 0],[1 0 0 1], [0.75, .75, .75])
  Toaster : Subsystem
      mask Display: image('toaster.jpg');
  Tv : Subsystem
      mask Display: image('Tel.jpg');
  Water tank : Subsystem
      mask Display: image('Hot Water.jpg');
  Connection Port : PMIOPort  Side=Left
  wires:
  Shower/1(Out1) -> Water tank/1(Mass flow rate)
  connections:
  Refrigerator/LConn1 -- Water tank/LConn1 -- Light 4/LConn1 -- Light 5/LConn1 -- Clock 1/LConn1 -- Lamp 1/LConn1 -- Clock 2/LConn1 -- Light 6/LConn1 -- Lamp 2/LConn1 -- Laptop_Charger/RConn1 -- Laptop_Charger/LConn1 -- Phone_Charger/RConn1 -- Phone_Charger/LConn2 -- Phone_Charger/LConn1 -- Light 7/LConn1 -- Lamp 3/LConn1 -- Stereo/LConn1 -- Tv/LConn1 -- Heater/LConn1 -- Kettle/LConn1 -- Light 2/LConn1 -- Toaster/LConn1 -- Light 1/LConn1 -- Oven/LConn1 -- Connection Port/RConn1

== system Microgrid_Library/House 7 S ==
  Clock 1 : Subsystem
      mask Display: image('Clock.jpg');
  Clock 2 : Subsystem
      mask Display: image('Clock.jpg');
  Kettle : Subsystem
      mask Display: image('jug.jpg');
  Lamp 1 : Subsystem
      mask Display: image('Lamp_1.jpg');
  Lamp 2 : Subsystem
      mask Display: image('Lamp_1.jpg');
  Lamp 3 : Subsystem
      mask Display: image('Lamp_1.jpg');
  Laptop_Charger : Subsystem
      mask Display: image('Laptop.jpg');
  Light 1 : Subsystem
      mask Display: image('LightBulb.jpg');
  Light 2 : Subsystem
      mask Display: image('Dishwasher.jpg');
  Light 4 : Subsystem
      mask Display: image('LightBulb.jpg');
  Light 5 : Subsystem
      mask Display: image('LightBulb.jpg');
  Light 6 : Subsystem
      mask Display: image('LightBulb.jpg');
  Light 7 : Subsystem
      mask Display: image('LightBulb.jpg');
  Oven : Subsystem
      mask Display: image('oven.jpg');
  Phone_Charger : Subsystem
      mask Display: image('Phone.jpg');
  Refrigerator : Subsystem
      mask Display: image('firdge1.JPG');
  Shower : Subsystem
      mask Display: image('Shower.jpg');
  Stereo : Subsystem
      mask Display: image('Stereo.jpg');
  Subsystem : Subsystem
      mask Display: patch([1 1 0 0],[1 0 0 1], [1, .4, .4])
  Subsystem1 : Subsystem
      mask Display: patch([1 1 0 0],[1 0 0 1], [0.6, .8, 1])
  Subsystem2 : Subsystem
      mask Display: patch([1 1 0 0],[1 0 0 1], [0.4, 1, .4])
  Subsystem3 : Subsystem
      mask Display: patch([1 1 0 0],[1 0 0 1], [0.8, 0.42, 0])
  Subsystem4 : Subsystem
      mask Display: patch([1 1 0 0],[1 0 0 1], [0.75, .75, .75])
  Toaster : Subsystem
      mask Display: image('toaster.jpg');
  Tv : Subsystem
      mask Display: image('Tel.jpg');
  Water tank : Subsystem
      mask Display: image('Hot Water.jpg');
  Connection Port : PMIOPort  Side=Left
  wires:
  Shower/1(Out1) -> Water tank/1(Mass flow rate)
  connections:
  Lamp 2/LConn1 -- Light 6/LConn1 -- Clock 2/LConn1 -- Lamp 1/LConn1 -- Clock 1/LConn1 -- Light 5/LConn1 -- Light 4/LConn1 -- Water tank/LConn1 -- Refrigerator/LConn1 -- Light 2/LConn1 -- Connection Port/RConn1 -- Oven/LConn1 -- Light 1/LConn1 -- Toaster/LConn1 -- Kettle/LConn1 -- Laptop_Charger/RConn1 -- Laptop_Charger/LConn1 -- Phone_Charger/RConn1 -- Phone_Charger/LConn2 -- Phone_Charger/LConn1 -- Light 7/LConn1 -- Lamp 3/LConn1 -- Stereo/LConn1 -- Tv/LConn1

== system Microgrid_Library/House 7 W ==
  Clock 1 : Subsystem
      mask Display: image('Clock.jpg');
  Clock 2 : Subsystem
      mask Display: image('Clock.jpg');
  Heater : Subsystem
      mask Display: image('oil_heater.jpg');
  Kettle : Subsystem
      mask Display: image('jug.jpg');
  Lamp 1 : Subsystem
      mask Display: image('Lamp_1.jpg');
  Lamp 2 : Subsystem
      mask Display: image('Lamp_1.jpg');
  Lamp 3 : Subsystem
      mask Display: image('Lamp_1.jpg');
  Laptop_Charger : Subsystem
      mask Display: image('Laptop.jpg');
  Light 1 : Subsystem
      mask Display: image('LightBulb.jpg');
  Light 2 : Subsystem
      mask Display: image('Dishwasher.jpg');
  Light 4 : Subsystem
      mask Display: image('LightBulb.jpg');
  Light 5 : Subsystem
      mask Display: image('LightBulb.jpg');
  Light 6 : Subsystem
      mask Display: image('LightBulb.jpg');
  Light 7 : Subsystem
      mask Display: image('LightBulb.jpg');
  Oven : Subsystem
      mask Display: image('oven.jpg');
  Phone_Charger : Subsystem
      mask Display: image('Phone.jpg');
  Refrigerator : Subsystem
      mask Display: image('firdge1.JPG');
  Shower : Subsystem
      mask Display: image('Shower.jpg');
  Stereo : Subsystem
      mask Display: image('Stereo.jpg');
  Subsystem : Subsystem
      mask Display: patch([1 1 0 0],[1 0 0 1], [1, .4, .4])
  Subsystem1 : Subsystem
      mask Display: patch([1 1 0 0],[1 0 0 1], [0.6, .8, 1])
  Subsystem2 : Subsystem
      mask Display: patch([1 1 0 0],[1 0 0 1], [0.4, 1, .4])
  Subsystem3 : Subsystem
      mask Display: patch([1 1 0 0],[1 0 0 1], [0.8, 0.42, 0])
  Subsystem4 : Subsystem
      mask Display: patch([1 1 0 0],[1 0 0 1], [0.75, .75, .75])
  Toaster : Subsystem
      mask Display: image('toaster.jpg');
  Tv : Subsystem
      mask Display: image('Tel.jpg');
  Water tank : Subsystem
      mask Display: image('Hot Water.jpg');
  Connection Port : PMIOPort  Side=Left
  wires:
  Shower/1(Out1) -> Water tank/1(Mass flow rate)
  connections:
  Connection Port/RConn1 -- Oven/LConn1 -- Light 1/LConn1 -- Toaster/LConn1 -- Light 2/LConn1 -- Kettle/LConn1 -- Water tank/LConn1 -- Heater/LConn1 -- Tv/LConn1 -- Stereo/LConn1 -- Lamp 3/LConn1 -- Light 7/LConn1 -- Phone_Charger/LConn1 -- Phone_Charger/LConn2 -- Phone_Charger/RConn1 -- Laptop_Charger/LConn1 -- Laptop_Charger/RConn1 -- Lamp 2/LConn1 -- Light 6/LConn1 -- Clock 2/LConn1 -- Lamp 1/LConn1 -- Clock 1/LConn1 -- Light 5/LConn1 -- Light 4/LConn1 -- Refrigerator/LConn1

== system Microgrid_Library/House 8 S ==
  Clock 1 : Subsystem
      mask Display: image('Clock.jpg');
  Clock 2 : Subsystem
      mask Display: image('Clock.jpg');
  Kettle : Subsystem
      mask Display: image('jug.jpg');
  Lamp 1 : Subsystem
      mask Display: image('Lamp_1.jpg');
  Lamp 2 : Subsystem
      mask Display: image('Lamp_1.jpg');
  Lamp 3 : Subsystem
      mask Display: image('Lamp_1.jpg');
  Laptop_Charger : Subsystem
      mask Display: image('Laptop.jpg');
  Light 1 : Subsystem
      mask Display: image('LightBulb.jpg');
  Light 2 : Subsystem
      mask Display: image('LightBulb.jpg');
  Light 3 : Subsystem
      mask Display: image('LightBulb.jpg');
  Light 4 : Subsystem
      mask Display: image('LightBulb.jpg');
  Light 5 : Subsystem
      mask Display: image('LightBulb.jpg');
  Light 6 : Subsystem
      mask Display: image('LightBulb.jpg');
  Oven : Subsystem
      mask Display: image('oven.jpg');
  Phone_Charger : Subsystem
      mask Display: image('Phone.jpg');
  Refrigerator : Subsystem
      mask Display: image('firdge1.JPG');
  Shower : Subsystem
      mask Display: image('Shower.jpg');
  Stereo : Subsystem
      mask Display: image('Stereo.jpg');
  Subsystem : Subsystem
      mask Display: patch([1 1 0 0],[1 0 0 1], [1, .4, .4])
  Subsystem1 : Subsystem
      mask Display: patch([1 1 0 0],[1 0 0 1], [0.6, .8, 1])
  Subsystem2 : Subsystem
      mask Display: patch([1 1 0 0],[1 0 0 1], [0.4, 1, .4])
  Subsystem3 : Subsystem
      mask Display: patch([1 1 0 0],[1 0 0 1], [0.8, 0.42, 0])
  Subsystem4 : Subsystem
      mask Display: patch([1 1 0 0],[1 0 0 1], [0.75, .75, .75])
  Toaster : Subsystem
      mask Display: image('toaster.jpg');
  Tv : Subsystem
      mask Display: image('Tel.jpg');
  Water tank : Subsystem
      mask Display: image('Hot Water.jpg');
  Connection Port : PMIOPort  Side=Left
  wires:
  Shower/1(Out1) -> Water tank/1(Mass flow rate)
  connections:
  Laptop_Charger/LConn1 -- Laptop_Charger/RConn1 -- Lamp 2/LConn1 -- Light 5/LConn1 -- Clock 2/LConn1 -- Lamp 1/LConn1 -- Clock 1/LConn1 -- Light 4/LConn1 -- Light 3/LConn1 -- Water tank/LConn1 -- Refrigerator/LConn1 -- Kettle/LConn1 -- Toaster/LConn1 -- Light 2/LConn1 -- Oven/LConn1 -- Connection Port/RConn1 -- Light 1/LConn1 -- Phone_Charger/RConn1 -- Phone_Charger/LConn2 -- Phone_Charger/LConn1 -- Light 6/LConn1 -- Lamp 3/LConn1 -- Stereo/LConn1 -- Tv/LConn1
